simulink model "TosAeb"  (R2021a)
settings: Solver=FixedStepDiscrete  StartTime=0.0  StopTime=inf  FixedStep=0.01  RelTol=1e-3
undefined symbols (not defined in the file or in its folder): ACCModSts, AccSts1, AEB_ObjectMovingOrientation, AEB_ObjectMovingState, CN_BTCActive, CN_CurrentGearR, CN_CurrentGearR_DHT, CN_PTCActive, CN_VDCActive, CN_VSC_EspOff, CN_VSC_FCW_AEB_Error, CN_VSC_FCW_AEB_NoDispay, CN_VSC_FCW_AEB_PerfmDgdtn, CN_VSC_FCW_AEB_TempUnAvlbl, CN_VSC_VehStandstill, CNaccelAvoidMax, CNaccelAvoidMin, CNbrakePedalPress, CNcomfortStrLeftAccelMax, CNcomfortStrLeftAccelMin, CNcomfortStrRightAccelMax, CNcomfortStrRightAccelMin, CNDrvDoorOpen, CNDrvModeEco, CNDrvModeNorm, CNDrvModeOther, CNDrvModeSport, CNDrvSBRreminder, CNNull, CNobjInvalidID, CNPassDoorOpen, CNPassSBRreminder, CNPEBSAccelNegInf, CNPEBSAccelNull, CNPEBSTimeInf, CNPEBSTimeNull, CNSTOPMAX, CNSTOPNULL, CNsubjWidth, CNSwtOFF, CNSwtON, CNTTCMAX, CNTTCNULL, CNVehConfigAEBCycNone, CNVehConfigAEBNone, CNVehConfigAEBPedNone, CNVehConfigFCWNone, CNVehConfigJANone, CollisionSeverity, FALSE, FCWSnvty, JACycObjCalcInfoDefaultParams, JAPedObjCalcInfoDefaultParams, JAVehObjCalcInfoDefaultParams, latCycObjCalcInfoDefaultParams, latPedObjCalcInfoDefaultParams, longObjCalcInfoDefaultParams, ModSts, movingStatus, ObjectType ... (+1112)
libraries: simulink x3031, AEBSFunctionLib x50, Ta_Library x33, AEBSStateMachineLib x23, Mylibrary x12, simulink_extras x7, ObjSelect_lib x3, dspstat3 x2
(part 28 of 102)

== system TosAeb/FcwAebJa_Step_sys/ALG_AEBSystem/ALG_AEBController/AEB_FuncImpManagementApp/FuncImpArbCalc/BrkReadyFuncImpArbCalc1/BrkFuncImpSuppCondCalc ==
  prefillModStsArb : Inport
  ABAModStsArb : Inport  Port=2
  pedPrefillModStsArb : Inport  Port=3
  cycPrefillModStsArb : Inport  Port=4
  JA_vehPrefillModStsArb : Inport  Port=5
  JA_PTW_PrefillModStsArb : Inport  Port=6
  JA_cycPrefillModStsArb : Inport  Port=7
  JA_pedPrefillModStsArb : Inport  Port=8
  brkFuncActiveCond : Inport  Port=9
  Bus Creator : BusCreator  Inputs=9
  Bus Selector : BusSelector  OutputSignals=brkFuncActiveCond
  Bus Selector1 : BusSelector  OutputSignals=AEBModStsArb.latAEBModStsArb.pedABAModStsArb
  Bus Selector10 : BusSelector  OutputSignals=ABAModStsArb,pedABAModStsArb
  Bus Selector2 : BusSelector  OutputSignals=brkFuncActiveCond,AEBModStsArb.vehAEBModStsArb.prefillModStsArb
  Bus Selector3 : BusSelector  OutputSignals=AEBModStsArb.vehAEBModStsArb.prefillModStsArb,AEBModStsArb.latAEBModStsArb.pedPrefillModStsArb,brkFuncActiveCond
  Bus Selector4 : BusSelector  OutputSignals=AEBModStsArb.vehAEBModStsArb.prefillModStsArb,AEBModStsArb.latAEBModStsArb.pedPrefillModStsArb,AEBModStsArb.latAEBModStsArb.cycPrefillModStsArb,brkFuncActiveCond
  Bus Selector5 : BusSelector  OutputSignals=prefillModStsArb,pedPrefillModStsArb,JA_PTW_PrefillModStsArb,JA_cycPrefillModStsArb,JA_pedPrefillModStsArb,brkFuncActiveCond
  Bus Selector6 : BusSelector  OutputSignals=prefillModStsArb,pedPrefillModStsArb,brkFuncActiveCond
  Bus Selector7 : BusSelector  OutputSignals=prefillModStsArb,pedPrefillModStsArb,JA_pedPrefillModStsArb,brkFuncActiveCond
  Bus Selector8 : BusSelector  OutputSignals=prefillModStsArb,pedPrefillModStsArb,JA_cycPrefillModStsArb,JA_pedPrefillModStsArb,brkFuncActiveCond
  Bus Selector9 : BusSelector  OutputSignals=prefillModStsArb,pedPrefillModStsArb,JA_cycPrefillModStsArb,JA_pedPrefillModStsArb,brkFuncActiveCond
  Compare To Constant{,2,4..5,7..8,10..15,17..21,23..24,26..28,30..32,34..35} (27 alike) : simulink/Logic and Bit Operations/Compare To Constant  relop===  const=ModSts.ActiveState  OutDataTypeStr=boolean
  Compare To Constant{1,3,6,9,16,22,25,29,33} (9 alike) : simulink/Logic and Bit Operations/Compare To Constant  relop===  const=TRUE  OutDataTypeStr=boolean
  Constant : Constant  Value=FALSE
  Logical Operator : Logic  Operator=OR  AllPortsSameDT=off  OutDataTypeStr=boolean
  Logical Operator1 : Logic  Operator=OR  Inputs=3  AllPortsSameDT=off  OutDataTypeStr=boolean
  Logical Operator2 : Logic  Operator=OR  Inputs=4  AllPortsSameDT=off  OutDataTypeStr=boolean
  Logical Operator3 : Logic  Operator=OR  Inputs=6  AllPortsSameDT=off  OutDataTypeStr=boolean
  Logical Operator4 : Logic  Operator=OR  Inputs=3  AllPortsSameDT=off  OutDataTypeStr=boolean
  Logical Operator5 : Logic  Operator=OR  Inputs=4  AllPortsSameDT=off  OutDataTypeStr=boolean
  Logical Operator6 : Logic  Operator=OR  Inputs=5  AllPortsSameDT=off  OutDataTypeStr=boolean
  Logical Operator7 : Logic  Operator=OR  Inputs=5  AllPortsSameDT=off  OutDataTypeStr=boolean
  Logical Operator8 : Logic  Operator=OR  AllPortsSameDT=off  OutDataTypeStr=boolean
  JA_PrefillFuncImpSuppCond : Outport
  cycPrefillFuncImpSuppCond : Outport  Port=2
  pedPrefillFuncImpSuppCond : Outport  Port=3
  prefillFuncImpSuppCond : Outport  Port=4
  ABAFuncImpSuppCond : Outport  Port=5
  pedABAFuncImpSuppCond : Outport  Port=6
  JA_vehPrefillFuncImpSuppCond : Outport  Port=7
  JA_pedPrefillFuncImpSuppCond : Outport  Port=8
  JA_cycPrefillFuncImpSuppCond : Outport  Port=9
  JA_PTW_PrefillFuncImpSuppCond : Outport  Port=10
  FCTB_PrefillFuncImpSuppCond : Outport  Port=11
  FCTB_ABAPrefillFuncImpSuppCond : Outport  Port=12
  wires:
  Bus Selector/1 -> Compare To Constant1/1  "<brkFuncActiveCond>"
  Compare To Constant1/1 -> prefillFuncImpSuppCond/1
  Bus Selector1/1 -> Compare To Constant/1  "<pedABAModStsArb>"
  Compare To Constant/1 -> ABAFuncImpSuppCond/1
  Bus Selector2/1 -> Compare To Constant3/1  "<brkFuncActiveCond>"
  Bus Selector2/2 -> Compare To Constant2/1  "<prefillModStsArb>"
  Compare To Constant3/1 -> Logical Operator/1
  Compare To Constant2/1 -> Logical Operator/2
  Logical Operator/1 -> pedPrefillFuncImpSuppCond/1
  Constant/1 -> pedABAFuncImpSuppCond/1
  Bus Selector3/1 -> Compare To Constant4/1  "<prefillModStsArb>"
  Bus Selector3/2 -> Compare To Constant5/1  "<pedPrefillModStsArb>"
  Bus Selector3/3 -> Compare To Constant6/1  "<brkFuncActiveCond>"
  Compare To Constant4/1 -> Logical Operator1/1
  Compare To Constant5/1 -> Logical Operator1/2
  Compare To Constant6/1 -> Logical Operator1/3
  Logical Operator1/1 -> cycPrefillFuncImpSuppCond/1
  Logical Operator2/1 -> JA_PrefillFuncImpSuppCond/1
  Compare To Constant9/1 -> Logical Operator2/4
  Compare To Constant8/1 -> Logical Operator2/2
  Compare To Constant7/1 -> Logical Operator2/1
  Bus Selector4/4 -> Compare To Constant9/1  "<brkFuncActiveCond>"
  Bus Selector4/2 -> Compare To Constant8/1  "<pedPrefillModStsArb>"
  Bus Selector4/1 -> Compare To Constant7/1  "<prefillModStsArb>"
  Bus Selector4/3 -> Compare To Constant10/1  "<cycPrefillModStsArb>"
  Compare To Constant10/1 -> Logical Operator2/3
  JA_pedPrefillModStsArb/1 -> Bus Creator/8  "JA_pedPrefillModStsArb"
  JA_PTW_PrefillModStsArb/1 -> Bus Creator/6  "JA_PTW_PrefillModStsArb"
  JA_cycPrefillModStsArb/1 -> Bus Creator/7  "JA_cycPrefillModStsArb"
  brkFuncActiveCond/1 -> Bus Creator/9  "brkFuncActiveCond"
  prefillModStsArb/1 -> Bus Creator/1  "prefillModStsArb"
  pedPrefillModStsArb/1 -> Bus Creator/3  "pedPrefillModStsArb"
  ABAModStsArb/1 -> Bus Creator/2  "ABAModStsArb"
  JA_vehPrefillModStsArb/1 -> Bus Creator/5  "JA_vehPrefillModStsArb"
  cycPrefillModStsArb/1 -> Bus Creator/4  "cycPrefillModStsArb"
  Compare To Constant31/1 -> Logical Operator7/3
  Compare To Constant30/1 -> Logical Operator7/2
  Bus Selector9/3 -> Compare To Constant31/1  "<JA_cycPrefillModStsArb>"
  Bus Selector9/5 -> Compare To Constant33/1  "<brkFuncActiveCond>"
  Bus Selector9/4 -> Compare To Constant32/1  "<JA_pedPrefillModStsArb>"
  Compare To Constant32/1 -> Logical Operator7/4
  Bus Selector5/1 -> Compare To Constant11/1  "<prefillModStsArb>"
  Compare To Constant12/1 -> Logical Operator3/2
  Bus Selector5/2 -> Compare To Constant12/1  "<pedPrefillModStsArb>"
  Logical Operator6/1 -> JA_PTW_PrefillFuncImpSuppCond/1
  Bus Selector8/5 -> Compare To Constant29/1  "<brkFuncActiveCond>"
  Bus Selector8/2 -> Compare To Constant23/1  "<pedPrefillModStsArb>"
  Bus Selector8/1 -> Compare To Constant21/1  "<prefillModStsArb>"
  Compare To Constant13/1 -> Logical Operator3/3
  Compare To Constant25/1 -> Logical Operator5/4
  Compare To Constant22/1 -> Logical Operator4/3
  Bus Creator/1 -> Bus Selector10/1, Bus Selector9/1, Bus Selector8/1, Bus Selector7/1, Bus Selector6/1, Bus Selector5/1, Bus Selector1/1, Bus Selector/1, Bus Selector2/1, Bus Selector3/1, Bus Selector4/1
  Compare To Constant11/1 -> Logical Operator3/1
  Bus Selector7/2 -> Compare To Constant20/1  "<pedPrefillModStsArb>"
  Bus Selector7/4 -> Compare To Constant25/1  "<brkFuncActiveCond>"
  Compare To Constant33/1 -> Logical Operator7/5
  Compare To Constant28/1 -> Logical Operator6/4
  Bus Selector6/3 -> Compare To Constant22/1  "<brkFuncActiveCond>"
  Compare To Constant20/1 -> Logical Operator5/2
  Compare To Constant14/1 -> Logical Operator3/4
  Logical Operator5/1 -> JA_cycPrefillFuncImpSuppCond/1
  Bus Selector5/5 -> Compare To Constant15/1  "<JA_pedPrefillModStsArb>"
  Bus Selector8/3 -> Compare To Constant27/1  "<JA_cycPrefillModStsArb>"
  Compare To Constant26/1 -> Logical Operator7/1
  Compare To Constant18/1 -> Logical Operator4/2
  Compare To Constant17/1 -> Logical Operator4/1
  Bus Selector5/3 -> Compare To Constant13/1  "<JA_PTW_PrefillModStsArb>"
  Compare To Constant21/1 -> Logical Operator6/1
  Compare To Constant15/1 -> Logical Operator3/5
  Bus Selector5/6 -> Compare To Constant16/1  "<brkFuncActiveCond>"
  Bus Selector7/3 -> Compare To Constant24/1  "<JA_pedPrefillModStsArb>"
  Compare To Constant23/1 -> Logical Operator6/2
  Bus Selector5/4 -> Compare To Constant14/1  "<JA_cycPrefillModStsArb>"
  Compare To Constant16/1 -> Logical Operator3/6
  Bus Selector8/4 -> Compare To Constant28/1  "<JA_pedPrefillModStsArb>"
  Compare To Constant29/1 -> Logical Operator6/5
  Bus Selector9/1 -> Compare To Constant26/1  "<prefillModStsArb>"
  Compare To Constant34/1 -> Logical Operator8/1
  Logical Operator8/1 -> FCTB_ABAPrefillFuncImpSuppCond/1
  Logical Operator7/1 -> FCTB_PrefillFuncImpSuppCond/1
  Compare To Constant35/1 -> Logical Operator8/2
  Logical Operator4/1 -> JA_pedPrefillFuncImpSuppCond/1
  Compare To Constant24/1 -> Logical Operator5/3
  Compare To Constant27/1 -> Logical Operator6/3
  Bus Selector10/2 -> Compare To Constant35/1  "<pedABAModStsArb>"
  Logical Operator3/1 -> JA_vehPrefillFuncImpSuppCond/1
  Bus Selector9/2 -> Compare To Constant30/1  "<pedPrefillModStsArb>"
  Bus Selector6/1 -> Compare To Constant17/1  "<prefillModStsArb>"
  Bus Selector7/1 -> Compare To Constant19/1  "<prefillModStsArb>"
  Bus Selector10/1 -> Compare To Constant34/1  "<ABAModStsArb>"
  Bus Selector6/2 -> Compare To Constant18/1  "<pedPrefillModStsArb>"
  Compare To Constant19/1 -> Logical Operator5/1

== system TosAeb/FcwAebJa_Step_sys/ALG_AEBSystem/ALG_AEBController/AEB_FuncImpManagementApp/FuncImpArbCalc/BrkReadyFuncImpArbCalc1/BrkReadyFuncActvCondCalc ==
  PrefillFuncImpArb : Inport
  ABAFuncImpArb : Inport  Port=2
  pedPrefillFuncImpArb : Inport  Port=3
  pedABAFuncImpArb : Inport  Port=4
  cycPrefillFuncImpArb : Inport  Port=5
  JA_vehPrefillFuncImpArb : Inport  Port=6
  Compare To Constant{,1..5} (6 alike) : simulink/Logic and Bit Operations/Compare To Constant  relop===  const=ModSts.ActiveState  OutDataTypeStr=boolean
  Logical Operator : Logic  Operator=OR  Inputs=6  AllPortsSameDT=off  OutDataTypeStr=boolean
  brkReadyActvCond : Outport
  wires:
  PrefillFuncImpArb/1 -> Compare To Constant/1
  ABAFuncImpArb/1 -> Compare To Constant1/1
  pedPrefillFuncImpArb/1 -> Compare To Constant2/1
  pedABAFuncImpArb/1 -> Compare To Constant3/1
  cycPrefillFuncImpArb/1 -> Compare To Constant4/1
  JA_vehPrefillFuncImpArb/1 -> Compare To Constant5/1
  Compare To Constant4/1 -> Logical Operator/5
  Compare To Constant5/1 -> Logical Operator/6
  Compare To Constant3/1 -> Logical Operator/4
  Compare To Constant2/1 -> Logical Operator/3
  Compare To Constant1/1 -> Logical Operator/2
  Compare To Constant/1 -> Logical Operator/1
  Logical Operator/1 -> brkReadyActvCond/1

== system TosAeb/FcwAebJa_Step_sys/ALG_AEBSystem/ALG_AEBController/AEB_FuncImpManagementApp/FuncImpArbCalc/BrkReadyFuncImpArbCalc1/BrkReadyFuncImpAborCondCalc ==
  prefillModStsArb : Inport
  ABAModStsArb : Inport  Port=2
  pedPrefillModStsArb : Inport  Port=3
  pedABAModStsArb : Inport  Port=4
  cycPrefillModStsArb : Inport  Port=5
  JA_vehPrefillModStsArb : Inport  Port=6
  JA_PTW_PrefillModStsArb : Inport  Port=7
  JA_cycPrefillModStsArb : Inport  Port=8
  JA_pedPrefillModStsArb : Inport  Port=9
  brkFuncActiveCond : Inport  Port=10
  Bus Creator : BusCreator  Inputs=10
  Bus Selector : BusSelector  OutputSignals=brkFuncActiveCond
  Bus Selector1 : BusSelector  OutputSignals=prefillModStsArb,brkFuncActiveCond
  Bus Selector10 : BusSelector  OutputSignals=ABAModStsArb,pedABAModStsArb
  Bus Selector2 : BusSelector  OutputSignals=prefillModStsArb,pedPrefillModStsArb,brkFuncActiveCond
  Bus Selector3 : BusSelector  OutputSignals=prefillModStsArb,pedPrefillModStsArb,cycPrefillModStsArb,brkFuncActiveCond
  Bus Selector4 : BusSelector  OutputSignals=pedPrefillModStsArb
  Bus Selector5 : BusSelector  OutputSignals=prefillModStsArb,pedPrefillModStsArb,JA_PTW_PrefillModStsArb,JA_cycPrefillModStsArb,JA_pedPrefillModStsArb,brkFuncActiveCond
  Bus Selector6 : BusSelector  OutputSignals=prefillModStsArb,pedPrefillModStsArb,brkFuncActiveCond
  Bus Selector7 : BusSelector  OutputSignals=prefillModStsArb,pedPrefillModStsArb,JA_pedPrefillModStsArb,brkFuncActiveCond
  Bus Selector8 : BusSelector  OutputSignals=prefillModStsArb,pedPrefillModStsArb,JA_cycPrefillModStsArb,JA_pedPrefillModStsArb,brkFuncActiveCond
  Bus Selector9 : BusSelector  OutputSignals=prefillModStsArb,pedPrefillModStsArb,JA_cycPrefillModStsArb,JA_pedPrefillModStsArb,brkFuncActiveCond
  Compare To Constant{,3,6,9,16,22,25,29,33} (9 alike) : simulink/Logic and Bit Operations/Compare To Constant  relop===  const=TRUE  OutDataTypeStr=boolean
  Compare To Constant{1..2,4..5,7..8,10..15,17..21,23..24,26..28,30..32,34..35} (27 alike) : simulink/Logic and Bit Operations/Compare To Constant  relop===  const=ModSts.ActiveState  OutDataTypeStr=boolean
  Constant : Constant  Value=FALSE
  Logical Operator : Logic  Operator=OR  AllPortsSameDT=off  OutDataTypeStr=boolean
  Logical Operator1 : Logic  Operator=OR  Inputs=3  AllPortsSameDT=off  OutDataTypeStr=boolean
  Logical Operator2 : Logic  Operator=OR  Inputs=4  AllPortsSameDT=off  OutDataTypeStr=boolean
  Logical Operator3 : Logic  Operator=OR  Inputs=6  AllPortsSameDT=off  OutDataTypeStr=boolean
  Logical Operator4 : Logic  Operator=OR  Inputs=3  AllPortsSameDT=off  OutDataTypeStr=boolean
  Logical Operator5 : Logic  Operator=OR  Inputs=4  AllPortsSameDT=off  OutDataTypeStr=boolean
  Logical Operator6 : Logic  Operator=OR  Inputs=5  AllPortsSameDT=off  OutDataTypeStr=boolean
  Logical Operator7 : Logic  Operator=OR  Inputs=5  AllPortsSameDT=off  OutDataTypeStr=boolean
  Logical Operator8 : Logic  Operator=OR  AllPortsSameDT=off  OutDataTypeStr=boolean
  JA_vehPrefillFuncImpAborCond : Outport
  cycPrefillFuncImpAborCond : Outport  Port=2
  pedPrefillFuncImpAborCond : Outport  Port=3
  prefillFuncImpAborCond : Outport  Port=4
  ABAFuncImpAborCond : Outport  Port=5
  pedABAFuncImpAborCond : Outport  Port=6
  JA_vehPrefillFuncImpAborArb : Outport  Port=7
  JA_pedPrefillFuncImpAborArb : Outport  Port=8
  JA_cycPrefillFuncImpAborArb : Outport  Port=9
  JA_PTW_PrefillFuncImpAborArb : Outport  Port=10
  FCTB_PrefillFuncImpAborArb : Outport  Port=11
  FCTB_ABAPrefillFuncImpAborArb : Outport  Port=12
  wires:
  Compare To Constant/1 -> prefillFuncImpAborCond/1
  Compare To Constant2/1 -> Logical Operator/1
  Compare To Constant3/1 -> Logical Operator/2
  Logical Operator/1 -> pedPrefillFuncImpAborCond/1
  Constant/1 -> pedABAFuncImpAborCond/1
  Compare To Constant4/1 -> Logical Operator1/1
  Compare To Constant6/1 -> Logical Operator1/3
  Compare To Constant5/1 -> Logical Operator1/2
  Logical Operator1/1 -> cycPrefillFuncImpAborCond/1
  Logical Operator2/1 -> JA_vehPrefillFuncImpAborCond/1
  Compare To Constant8/1 -> Logical Operator2/2
  Compare To Constant9/1 -> Logical Operator2/4
  Compare To Constant7/1 -> Logical Operator2/1
  Compare To Constant10/1 -> Logical Operator2/3
  ABAModStsArb/1 -> Bus Creator/2  "ABAModStsArb"
  prefillModStsArb/1 -> Bus Creator/1  "prefillModStsArb"
  pedPrefillModStsArb/1 -> Bus Creator/3  "pedPrefillModStsArb"
  cycPrefillModStsArb/1 -> Bus Creator/5  "cycPrefillModStsArb"
  JA_vehPrefillModStsArb/1 -> Bus Creator/6  "JA_vehPrefillModStsArb"
  brkFuncActiveCond/1 -> Bus Creator/10  "brkFuncActiveCond"
  Bus Selector/1 -> Compare To Constant/1  "<brkFuncActiveCond>"
  Bus Selector1/1 -> Compare To Constant2/1  "<prefillModStsArb>"
  Bus Selector1/2 -> Compare To Constant3/1  "<brkFuncActiveCond>"
  Bus Selector2/3 -> Compare To Constant6/1  "<brkFuncActiveCond>"
  Bus Selector2/2 -> Compare To Constant5/1  "<pedPrefillModStsArb>"
  Bus Selector2/1 -> Compare To Constant4/1  "<prefillModStsArb>"
  Bus Selector3/2 -> Compare To Constant8/1  "<pedPrefillModStsArb>"
  Bus Selector3/1 -> Compare To Constant7/1  "<prefillModStsArb>"
  Bus Selector3/3 -> Compare To Constant10/1  "<cycPrefillModStsArb>"
  Bus Selector3/4 -> Compare To Constant9/1  "<brkFuncActiveCond>"
  Bus Selector4/1 -> Compare To Constant11/1  "<pedPrefillModStsArb>"
  Compare To Constant11/1 -> ABAFuncImpAborCond/1
  JA_PTW_PrefillModStsArb/1 -> Bus Creator/7  "JA_PTW_PrefillModStsArb"
  JA_cycPrefillModStsArb/1 -> Bus Creator/8  "JA_cycPrefillModStsArb"
  JA_pedPrefillModStsArb/1 -> Bus Creator/9  "JA_pedPrefillModStsArb"
  pedABAModStsArb/1 -> Bus Creator/4  "pedABAModStsArb"
  Bus Selector5/1 -> Compare To Constant1/1  "<prefillModStsArb>"
  Bus Selector5/2 -> Compare To Constant12/1  "<pedPrefillModStsArb>"
  Bus Selector5/3 -> Compare To Constant13/1  "<JA_PTW_PrefillModStsArb>"
  Bus Selector5/4 -> Compare To Constant14/1  "<JA_cycPrefillModStsArb>"
  Bus Selector5/5 -> Compare To Constant15/1  "<JA_pedPrefillModStsArb>"
  Bus Selector5/6 -> Compare To Constant16/1  "<brkFuncActiveCond>"
  Compare To Constant16/1 -> Logical Operator3/6
  Compare To Constant15/1 -> Logical Operator3/5
  Compare To Constant14/1 -> Logical Operator3/4
  Compare To Constant13/1 -> Logical Operator3/3
  Compare To Constant12/1 -> Logical Operator3/2
  Compare To Constant1/1 -> Logical Operator3/1
  Logical Operator3/1 -> JA_vehPrefillFuncImpAborArb/1
  Logical Operator4/1 -> JA_pedPrefillFuncImpAborArb/1
  Compare To Constant17/1 -> Logical Operator4/1
  Compare To Constant18/1 -> Logical Operator4/2
  Compare To Constant22/1 -> Logical Operator4/3
  Bus Selector6/3 -> Compare To Constant22/1  "<brkFuncActiveCond>"
  Bus Selector6/2 -> Compare To Constant18/1  "<pedPrefillModStsArb>"
  Bus Selector6/1 -> Compare To Constant17/1  "<prefillModStsArb>"
  Logical Operator5/1 -> JA_cycPrefillFuncImpAborArb/1
  Compare To Constant19/1 -> Logical Operator5/1
  Compare To Constant20/1 -> Logical Operator5/2
  Compare To Constant24/1 -> Logical Operator5/3
  Compare To Constant25/1 -> Logical Operator5/4
  Bus Selector7/4 -> Compare To Constant25/1  "<brkFuncActiveCond>"
  Bus Selector7/3 -> Compare To Constant24/1  "<JA_pedPrefillModStsArb>"
  Bus Selector7/2 -> Compare To Constant20/1  "<pedPrefillModStsArb>"
  Bus Selector7/1 -> Compare To Constant19/1  "<prefillModStsArb>"
  Compare To Constant27/1 -> Logical Operator6/3
  Bus Selector8/1 -> Compare To Constant21/1  "<prefillModStsArb>"
  Compare To Constant23/1 -> Logical Operator6/2
  Bus Selector8/2 -> Compare To Constant23/1  "<pedPrefillModStsArb>"
  Bus Selector8/3 -> Compare To Constant27/1  "<JA_cycPrefillModStsArb>"
  Compare To Constant21/1 -> Logical Operator6/1
  Compare To Constant29/1 -> Logical Operator6/5
  Bus Selector8/4 -> Compare To Constant28/1  "<JA_pedPrefillModStsArb>"
  Bus Selector8/5 -> Compare To Constant29/1  "<brkFuncActiveCond>"
  Compare To Constant28/1 -> Logical Operator6/4
  Logical Operator6/1 -> JA_PTW_PrefillFuncImpAborArb/1
  Logical Operator7/1 -> FCTB_PrefillFuncImpAborArb/1
  Compare To Constant32/1 -> Logical Operator7/4
  Bus Selector9/5 -> Compare To Constant33/1  "<brkFuncActiveCond>"
  Bus Selector9/4 -> Compare To Constant32/1  "<JA_pedPrefillModStsArb>"
  Compare To Constant33/1 -> Logical Operator7/5
  Compare To Constant26/1 -> Logical Operator7/1
  Bus Selector9/3 -> Compare To Constant31/1  "<JA_cycPrefillModStsArb>"
  Bus Selector9/2 -> Compare To Constant30/1  "<pedPrefillModStsArb>"
  Compare To Constant30/1 -> Logical Operator7/2
  Bus Selector9/1 -> Compare To Constant26/1  "<prefillModStsArb>"
  Compare To Constant31/1 -> Logical Operator7/3
  Bus Creator/1 -> Bus Selector10/1, Bus Selector9/1, Bus Selector8/1, Bus Selector7/1, Bus Selector6/1, Bus Selector5/1, Bus Selector4/1, Bus Selector/1, Bus Selector1/1, Bus Selector2/1, Bus Selector3/1
  Bus Selector10/1 -> Compare To Constant34/1  "<ABAModStsArb>"
  Bus Selector10/2 -> Compare To Constant35/1  "<pedABAModStsArb>"
  Compare To Constant35/1 -> Logical Operator8/2
  Compare To Constant34/1 -> Logical Operator8/1
  Logical Operator8/1 -> FCTB_ABAPrefillFuncImpAborArb/1

== system TosAeb/FcwAebJa_Step_sys/ALG_AEBSystem/ALG_AEBController/AEB_FuncImpManagementApp/FuncImpArbCalc/BrkReadyFuncImpArbCalc1/BrkReadyFuncImpArbCalc ==
  prefillModStsArb : Inport
  ABAModStsArb : Inport  Port=2
  pedPrefillModStsArb : Inport  Port=3
  cycPrefillModStsArb : Inport  Port=4
  JA_vehPrefillModStsArb : Inport  Port=5
  JA_PTW_PrefillModStsArb : Inport  Port=6
  JA_cycPrefillModStsArb : Inport  Port=7
  JA_pedPrefillModStsArb : Inport  Port=8
  JA_vehPrefillFuncImpAborCond : Inport  Port=9
  cycPrefillFuncImpAborCond : Inport  Port=10
  pedPrefillFuncImpAborCond : Inport  Port=11
  vehPrefillFuncImpAborCond : Inport  Port=12
  vehABAFuncImpAborCond : Inport  Port=13
  pedABAFuncImpAborCond : Inport  Port=14
  JA_vehPrefillFuncImpAborArb : Inport  Port=15
  JA_pedPrefillFuncImpAborArb : Inport  Port=16
  JA_cycPrefillFuncImpAborArb : Inport  Port=17
  JA_PTW_PrefillFuncImpAborArb : Inport  Port=18
  FCTB_PrefillFuncImpAborArb : Inport  Port=19
  FCTB_ABAPrefillFuncImpAborArb : Inport  Port=20
  ABAFuncImpArbCalc : Subsystem
  Bus Creator : BusCreator  Inputs=2
  Bus Creator4 : BusCreator  Inputs=8
  Bus Selector : BusSelector  OutputSignals=AEBModStsArb.vehAEBModStsArb.prefillModStsArb,brkReadyFuncImpAborCond.vehPrefillFuncImpAborCond
  Bus Selector1 : BusSelector  OutputSignals=AEBModStsArb.vehAEBModStsArb.ABAModStsArb,brkReadyFuncImpAborCond.vehABAFuncImpAborCond
  Bus Selector2 : BusSelector  OutputSignals=AEBModStsArb.latAEBModStsArb.pedPrefillModStsArb,brkReadyFuncImpAborCond.pedPrefillFuncImpAborCond
  Bus Selector3 : BusSelector  OutputSignals=AEBModStsArb.latAEBModStsArb.pedABAModStsArb,brkReadyFuncImpAborCond.pedABAFuncImpAborCond
  Bus Selector4 : BusSelector  OutputSignals=AEBModStsArb.latAEBModStsArb.cycPrefillModStsArb,brkReadyFuncImpAborCond.cycPrefillFuncImpAborCond
  Bus Selector5 : BusSelector  OutputSignals=AEBModStsArb.JA_vehAEBModStsArb.JA_vehPrefillModStsArb,brkReadyFuncImpAborCond.JA_vehPrefillFuncImpAborCond
  JA_vehPrefillFuncImpArbCalc : Subsystem
  PrefillFuncImpArbCalc : Subsystem
  cycPrefillFuncImpArbCalc : Subsystem
  pedABAFuncImpArbCalc : Subsystem
  pedPrefillFuncImpArbCalc : Subsystem
  prefillFuncImpArb : Outport
  ABAFuncImpArb : Outport  Port=2
  pedPrefillFuncImpArb : Outport  Port=3
  pedABAFuncImpArb : Outport  Port=4
  cycPrefillFuncImpArb : Outport  Port=5
  JA_vehPrefillFuncImpArb : Outport  Port=6
  wires:
  Bus Creator4/1 -> Bus Creator/1  "AEBModStsArb"
  Bus Creator/1 -> Bus Selector5/1, Bus Selector4/1, Bus Selector3/1, Bus Selector2/1, Bus Selector1/1, Bus Selector/1
  Bus Selector/1 -> PrefillFuncImpArbCalc/1(prefillModStsArb)  "<prefillModStsArb>"
  Bus Selector/2 -> PrefillFuncImpArbCalc/2(prefillFuncImpAborCon d)  "<vehPrefillFuncImpAborCond>"
  Bus Selector1/1 -> ABAFuncImpArbCalc/1(ABAModStsArb)  "<ABAModStsArb>"
  Bus Selector1/2 -> ABAFuncImpArbCalc/2(ABAFuncImpAborCond)  "<vehABAFuncImpAborCond>"
  Bus Selector2/1 -> pedPrefillFuncImpArbCalc/1(pedPrefillModStsArb)  "<pedPrefillModStsArb>"
  Bus Selector2/2 -> pedPrefillFuncImpArbCalc/2(pedPrefillFuncImpAborCond)  "<pedPrefillFuncImpAborCond>"
  Bus Selector3/1 -> pedABAFuncImpArbCalc/1(pedABAModStsArb)  "<pedABAModStsArb>"
  Bus Selector3/2 -> pedABAFuncImpArbCalc/2(pedABAFuncImpAborCond)  "<pedABAFuncImpAborCond>"
  Bus Selector4/1 -> cycPrefillFuncImpArbCalc/1(cycPrefillModStsArb)  "<cycPrefillModStsArb>"
  Bus Selector4/2 -> cycPrefillFuncImpArbCalc/2(cycPrefillFuncImpAborCond)  "<cycPrefillFuncImpAborCond>"
  Bus Selector5/1 -> JA_vehPrefillFuncImpArbCalc/1(JA_vehPrefillModStsArb)  "<JA_vehPrefillModStsArb>"
  Bus Selector5/2 -> JA_vehPrefillFuncImpArbCalc/2(JA_vehPrefillFuncImpAborCond)  "<JA_vehPrefillFuncImpAborCond>"
  PrefillFuncImpArbCalc/1(prefillFuncImpArb) -> prefillFuncImpArb/1  "prefillFuncImpArb"
  ABAFuncImpArbCalc/1(ABAFuncImpArb) -> ABAFuncImpArb/1  "ABAFuncImpArb"
  JA_vehPrefillFuncImpArbCalc/1(JA_vehPrefillFuncImpArb) -> JA_vehPrefillFuncImpArb/1  "JA_vehPrefillFuncImpArb"
  cycPrefillFuncImpArbCalc/1(cycPrefillFuncImpArb) -> cycPrefillFuncImpArb/1  "cycPrefillFuncImpArb"
  pedABAFuncImpArbCalc/1(pedABAFuncImpArb) -> pedABAFuncImpArb/1  "pedABAFuncImpArb"
  pedPrefillFuncImpArbCalc/1(pedPrefillFuncImpArb) -> pedPrefillFuncImpArb/1  "pedPrefillFuncImpArb"
  JA_cycPrefillModStsArb/1 -> Bus Creator4/7  "JA_cycPrefillModStsArb"
  prefillModStsArb/1 -> Bus Creator4/1  "prefillModStsArb"
  JA_vehPrefillModStsArb/1 -> Bus Creator4/5  "JA_vehPrefillModStsArb"
  pedPrefillModStsArb/1 -> Bus Creator4/3  "pedPrefillModStsArb"
  ABAModStsArb/1 -> Bus Creator4/2  "ABAModStsArb"
  JA_PTW_PrefillModStsArb/1 -> Bus Creator4/6  "JA_PTW_PrefillModStsArb"
  cycPrefillModStsArb/1 -> Bus Creator4/4  "cycPrefillModStsArb"
  JA_pedPrefillModStsArb/1 -> Bus Creator4/8  "JA_pedPrefillModStsArb"

== system TosAeb/FcwAebJa_Step_sys/ALG_AEBSystem/ALG_AEBController/AEB_FuncImpManagementApp/FuncImpArbCalc/WarningFuncImpArbCalc/WarningFuncImpAborCond ==
  AEBModStsArb : Inport
  brkReadyFuncActvCond : Inport  Port=2
  brkFuncActvCond : Inport  Port=3
  Bus Creator : BusCreator  Inputs=3
  Bus Selector : BusSelector  OutputSignals=brkReadyFuncActvCond,brkFuncActvCond,AEBModStsArb.vehAEBModStsArb.preWarningModStsArb,AEBModStsArb.vehAEBModStsArb.acuteWarningModStsArb,AEBModStsArb.latAEBModStsArb.pedPreWarningModStsArb
  Bus Selector1 : BusSelector  OutputSignals=AEBModStsArb.latAEBModStsArb.pedAEBfullModStsArb,AEBModStsArb.latAEBModStsArb.cycAEBfullModStsArb,AEBModStsArb.vehAEBModStsArb.EBAModStsArb
  Bus Selector2 : BusSelector  OutputSignals=brkFuncActvCond
  Bus Selector3 : BusSelector  OutputSignals=AEBModStsArb.vehAEBModStsArb.preWarningModStsArb,AEBModStsArb.latAEBModStsArb.cycAEBfullModStsArb,AEBModStsArb.vehAEBModStsArb.EBAModStsArb,AEBModStsArb.vehAEBModStsArb.AEBfullModStsArb
  Compare To Constant1 : simulink/Logic and Bit Operations/Compare To Constant  relop===  const=TRUE  OutDataTypeStr=boolean
  Compare To Constant{2,5..11,13..14} (10 alike) : simulink/Logic and Bit Operations/Compare To Constant  relop===  const=ModSts.ActiveState  OutDataTypeStr=boolean
  Compare To Constant3 : simulink/Logic and Bit Operations/Compare To Constant  relop===  const=TRUE  OutDataTypeStr=boolean
  Compare To Constant4 : simulink/Logic and Bit Operations/Compare To Constant  relop===  const=TRUE  OutDataTypeStr=boolean
  Logical Operator : Logic  Operator=OR  Inputs=5  AllPortsSameDT=off  OutDataTypeStr=boolean
  Logical Operator1 : Logic  Operator=OR  Inputs=3  AllPortsSameDT=off  OutDataTypeStr=boolean
  Logical Operator2 : Logic  Operator=OR  Inputs=4  AllPortsSameDT=off  OutDataTypeStr=boolean
  latentWarningFuncImpAborCond : Outport
  pedWarningFuncImpAborCond : Outport  Port=2
  preWarningFuncImpAborCond : Outport  Port=3
  acuteWarningFuncImpAborCond : Outport  Port=4
  wires:
  Compare To Constant4/1 -> acuteWarningFuncImpAborCond/1
  Bus Selector2/1 -> Compare To Constant4/1  "<brkFuncActvCond>"
  Logical Operator2/1 -> pedWarningFuncImpAborCond/1
  Compare To Constant3/1 -> Logical Operator/1
  Bus Selector/3 -> Compare To Constant2/1  "<preWarningModStsArb>"
  Bus Selector/4 -> Compare To Constant5/1  "<acuteWarningModStsArb>"
  Bus Selector/1 -> Compare To Constant3/1  "<brkReadyFuncActvCond>"
  Logical Operator/1 -> latentWarningFuncImpAborCond/1
  Compare To Constant2/1 -> Logical Operator/3
  Compare To Constant5/1 -> Logical Operator/4
  Compare To Constant6/1 -> Logical Operator/5
  Bus Selector/5 -> Compare To Constant6/1  "<pedPreWarningModStsArb>"
  Compare To Constant8/1 -> Logical Operator1/3
  Compare To Constant9/1 -> Logical Operator1/1
  Bus Selector1/2 -> Compare To Constant7/1  "<cycAEBfullModStsArb>"
  Bus Selector1/3 -> Compare To Constant8/1  "<EBAModStsArb>"
  Compare To Constant7/1 -> Logical Operator1/2
  Bus Selector1/1 -> Compare To Constant9/1  "<pedAEBfullModStsArb>"
  Bus Selector/2 -> Compare To Constant1/1  "<brkFuncActvCond>"
  Bus Selector3/3 -> Compare To Constant14/1  "<EBAModStsArb>"
  Bus Selector3/2 -> Compare To Constant13/1  "<cycAEBfullModStsArb>"
  Bus Selector3/4 -> Compare To Constant10/1  "<AEBfullModStsArb>"
  Compare To Constant11/1 -> Logical Operator2/1
  Compare To Constant13/1 -> Logical Operator2/2
  Compare To Constant14/1 -> Logical Operator2/3
  Bus Selector3/1 -> Compare To Constant11/1  "<preWarningModStsArb>"
  Compare To Constant10/1 -> Logical Operator2/4
  AEBModStsArb/1 -> Bus Creator/1  "AEBModStsArb"
  Compare To Constant1/1 -> Logical Operator/2
  brkReadyFuncActvCond/1 -> Bus Creator/2  "brkReadyFuncActvCond"
  brkFuncActvCond/1 -> Bus Creator/3  "brkFuncActvCond"
  Logical Operator1/1 -> preWarningFuncImpAborCond/1
  Bus Creator/1 -> Bus Selector/1, Bus Selector3/1, Bus Selector1/1, Bus Selector2/1

== system TosAeb/FcwAebJa_Step_sys/ALG_AEBSystem/ALG_AEBController/AEB_FuncImpManagementApp/FuncImpArbCalc/WarningFuncImpArbCalc/WarningFuncImpArbCalc ==
  AEBModStsArb : Inport
  warningFuncImpAborCondCond : Inport  Port=2
  AcuteFuncImpArbCalc : Subsystem
  Bus Creator : BusCreator  Inputs=2
  Bus Selector : BusSelector  OutputSignals=AEBModStsArb.vehAEBModStsArb.latentWarningModStsArb,warningFuncImpAborCond.vehLatentWarningFuncImpAborCond
  Bus Selector1 : BusSelector  OutputSignals=AEBModStsArb.vehAEBModStsArb.preWarningModStsArb,warningFuncImpAborCond.vehPreWarningFuncImpAborCond
  Bus Selector2 : BusSelector  OutputSignals=AEBModStsArb.vehAEBModStsArb.acuteWarningModStsArb,warningFuncImpAborCond.vehAcuteWarningFuncimpAborCond
  Bus Selector3 : BusSelector  OutputSignals=AEBModStsArb.latAEBModStsArb.pedPreWarningModStsArb,warningFuncImpAborCond.pedPreWarningFuncImpAborCond
  LatentWarningFuncImpArbCalc : Subsystem
  PreWarningFuncImpArbCalc : Subsystem
  pedPreWarningFuncImpArbCalc : Subsystem
  latentWarningFuncImpArb : Outport
  preWarningFuncImpArb : Outport  Port=2
  acuteWarningFuncImpArb : Outport  Port=3
  pedPreWarningFuncImpArb : Outport  Port=4
  wires:
  pedPreWarningFuncImpArbCalc/1(pedPreWarningFuncImpArb) -> pedPreWarningFuncImpArb/1  "pedPreWarningFuncImpArb"
  AcuteFuncImpArbCalc/1(acuteWarningFuncImpArb) -> acuteWarningFuncImpArb/1  "acuteWarningFuncImpArb"
  PreWarningFuncImpArbCalc/1(preWarningFuncImpArb) -> preWarningFuncImpArb/1  "preWarningFuncImpArb"
  LatentWarningFuncImpArbCalc/1(latentWarningFuncImpArb) -> latentWarningFuncImpArb/1  "latentWarningFuncImpArb"
  Bus Selector3/1 -> pedPreWarningFuncImpArbCalc/1(pedPreWarningModStsArb)  "<pedPreWarningModStsArb>"
  Bus Selector3/2 -> pedPreWarningFuncImpArbCalc/2(pedPreWarningFuncImpAborCond)  "<pedPreWarningFuncImpAborCond>"
  Bus Selector2/2 -> AcuteFuncImpArbCalc/2(acuteWarningFuncImpAborCond)  "<vehAcuteWarningFuncimpAborCond>"
  Bus Selector2/1 -> AcuteFuncImpArbCalc/1(acuteWarningModStsArb)  "<acuteWarningModStsArb>"
  Bus Selector1/1 -> PreWarningFuncImpArbCalc/1(preWarningModStsArb)  "<preWarningModStsArb>"
  Bus Selector1/2 -> PreWarningFuncImpArbCalc/2(preWarningFuncImpAborCond)  "<vehPreWarningFuncImpAborCond>"
  Bus Selector/2 -> LatentWarningFuncImpArbCalc/2(latentWarningFuncImpAborCond)  "<vehLatentWarningFuncImpAborCond>"
  Bus Selector/1 -> LatentWarningFuncImpArbCalc/1(latentWarningModStsArb)  "<latentWarningModStsArb>"
  Bus Creator/1 -> Bus Selector/1, Bus Selector1/1, Bus Selector2/1, Bus Selector3/1
  warningFuncImpAborCondCond/1 -> Bus Creator/2  "warningFuncImpAborCond"
  AEBModStsArb/1 -> Bus Creator/1  "AEBModStsArb"

== system TosAeb/FcwAebJa_Step_sys/ALG_AEBSystem/ALG_AEBController/AEB_FuncImpManagementApp/FuncImpArbCalc/WarningFuncImpArbCalc/WarningFuncImpSuppCond ==
  brkFuncActvCond : Inport
  brkReadyFuncActvCond : Inport  Port=2
  AEBModStsArb : Inport  Port=3
  Bus Creator : BusCreator  Inputs=3
  Bus Selector : BusSelector  OutputSignals=brkReadyFuncActvCond,brkFuncActvCond,AEBModStsArb.vehAEBModStsArb.preWarningModStsArb,AEBModStsArb.vehAEBModStsArb.acuteWarningModStsArb,AEBModStsArb.latAEBModStsArb.pedPreWarningModStsArb
  Bus Selector2 : BusSelector  OutputSignals=brkFuncActvCond
  Bus Selector3 : BusSelector  OutputSignals=brkFuncActvCond,AEBModStsArb.vehAEBModStsArb.preWarningModStsArb,AEBModStsArb.vehAEBModStsArb.acuteWarningModStsArb
  Compare To Constant{1,3..4,11} (4 alike) : simulink/Logic and Bit Operations/Compare To Constant  relop===  const=TRUE  OutDataTypeStr=boolean
  Compare To Constant{2,5..6,13..14} (5 alike) : simulink/Logic and Bit Operations/Compare To Constant  relop===  const=ModSts.ActiveState  OutDataTypeStr=boolean
  Logical Operator : Logic  Operator=OR  Inputs=5  AllPortsSameDT=off  OutDataTypeStr=boolean
  Logical Operator2 : Logic  Operator=OR  Inputs=3  AllPortsSameDT=off  OutDataTypeStr=boolean
  latentWarningFuncImpSuppCond : Outport
  pedWarningFuncImpSuppCond : Outport  Port=2
  preWarningFuncImpSuppCond : Outport  Port=3
  acuteWarningFuncImpSuppCond : Outport  Port=4
  wires:
  Bus Selector3/1 -> Compare To Constant11/1  "<brkFuncActvCond>"
  Bus Selector3/2 -> Compare To Constant13/1  "<preWarningModStsArb>"
  Bus Selector3/3 -> Compare To Constant14/1  "<acuteWarningModStsArb>"
  AEBModStsArb/1 -> Bus Creator/3  "AEBModStsArb"
  brkReadyFuncActvCond/1 -> Bus Creator/2  "brkReadyFuncActvCond"
  brkFuncActvCond/1 -> Bus Creator/1  "brkFuncActvCond"
  Bus Creator/1 -> Bus Selector3/1, Bus Selector2/1, Bus Selector/1
  Compare To Constant1/1 -> Logical Operator/2
  Compare To Constant14/1 -> Logical Operator2/3
  Compare To Constant13/1 -> Logical Operator2/2
  Compare To Constant11/1 -> Logical Operator2/1
  Bus Selector/2 -> Compare To Constant1/1  "<brkFuncActvCond>"
  Bus Selector/5 -> Compare To Constant6/1  "<pedPreWarningModStsArb>"
  Compare To Constant6/1 -> Logical Operator/5
  Compare To Constant5/1 -> Logical Operator/4
  Compare To Constant2/1 -> Logical Operator/3
  Logical Operator/1 -> latentWarningFuncImpSuppCond/1
  Bus Selector/1 -> Compare To Constant3/1  "<brkReadyFuncActvCond>"
  Bus Selector/4 -> Compare To Constant5/1  "<acuteWarningModStsArb>"
  Bus Selector/3 -> Compare To Constant2/1  "<preWarningModStsArb>"
  Compare To Constant3/1 -> Logical Operator/1
  Logical Operator2/1 -> pedWarningFuncImpSuppCond/1
  Bus Selector2/1 -> Compare To Constant4/1  "<brkFuncActvCond>"
  Compare To Constant4/1 -> preWarningFuncImpSuppCond/1, acuteWarningFuncImpSuppCond/1

== system TosAeb/FcwAebJa_Step_sys/ALG_AEBSystem/ALG_AEBController/AEB_FuncImpManagementApp/FuncImpArbCalc/WarningFuncImpArbCalc/warningFuncActvCondCalc ==
  latentWarningFuncImpArb : Inport
  preWarningFuncImpArb : Inport  Port=2
  acuteWarningFuncImpArb : Inport  Port=3
  pedPreWarningFuncImpArb : Inport  Port=4
  Compare To Constant{,1..3} (4 alike) : simulink/Logic and Bit Operations/Compare To Constant  relop===  const=ModSts.ActiveState  OutDataTypeStr=boolean
  Logical Operator : Logic  Operator=OR  Inputs=4  AllPortsSameDT=off  OutDataTypeStr=boolean
  warningFuncActvCond : Outport
  wires:
  Logical Operator/1 -> warningFuncActvCond/1
  Compare To Constant3/1 -> Logical Operator/4
  Compare To Constant2/1 -> Logical Operator/3
  Compare To Constant1/1 -> Logical Operator/2
  Compare To Constant/1 -> Logical Operator/1
  pedPreWarningFuncImpArb/1 -> Compare To Constant3/1
  acuteWarningFuncImpArb/1 -> Compare To Constant2/1
  preWarningFuncImpArb/1 -> Compare To Constant1/1
  latentWarningFuncImpArb/1 -> Compare To Constant/1

== system TosAeb/FcwAebJa_Step_sys/ALG_AEBSystem/ALG_AEBController/AEB_FuncImpManagementApp/FuncManeuArbCalc/BrkReadyManeuArbCalc/BrkReadyManeuAbor ==
  ABPTrigger : Inport
  ABATrigger : Inport  Port=2
  ABPActive : Inport  Port=3
  ABAActive : Inport  Port=4
  Compare To Constant : simulink/Logic and Bit Operations/Compare To Constant  relop===  const=TRUE  OutDataTypeStr=boolean
  Compare To Constant1 : simulink/Logic and Bit Operations/Compare To Constant  relop===  const=FALSE  OutDataTypeStr=boolean
  Compare To Constant2 : simulink/Logic and Bit Operations/Compare To Constant  relop===  const=TRUE  OutDataTypeStr=boolean
  Compare To Constant3 : simulink/Logic and Bit Operations/Compare To Constant  relop===  const=FALSE  OutDataTypeStr=boolean
  Logical Operator : Logic  Inputs=3  AllPortsSameDT=off  OutDataTypeStr=boolean
  Logical Operator1 : Logic  Inputs=3  AllPortsSameDT=off  OutDataTypeStr=boolean
  Unit Delay : UnitDelay  SampleTime=-1  HasFrameUpgradeWarning=on
  Unit Delay1 : UnitDelay  SampleTime=-1  HasFrameUpgradeWarning=on
  ABPFuncManeuAbor : Outport
  ABAFuncManeuAbor : Outport  Port=2
  wires:
  Logical Operator1/1 -> ABAFuncManeuAbor/1  "ABAFuncOptAbor"
  Logical Operator/1 -> ABPFuncManeuAbor/1  "ABPFuncOptAbor"
  ABAActive/1 -> Unit Delay1/1, Compare To Constant3/1
  Unit Delay1/1 -> Compare To Constant2/1
  ABATrigger/1 -> Logical Operator1/1
  Compare To Constant3/1 -> Logical Operator1/2
  Compare To Constant2/1 -> Logical Operator1/3
  Compare To Constant/1 -> Logical Operator/3
  Compare To Constant1/1 -> Logical Operator/2
  ABPTrigger/1 -> Logical Operator/1
  Unit Delay/1 -> Compare To Constant/1
  ABPActive/1 -> Compare To Constant1/1, Unit Delay/1

== system TosAeb/FcwAebJa_Step_sys/ALG_AEBSystem/ALG_AEBController/AEB_FuncImpManagementApp/FuncManeuArbCalc/BrkReadyManeuArbCalc/BrkReadyTriggerJudge ==
  breakReadyFuncImpArb : Inport
  ABPAvailable : Inport  Port=2
  ABAAvailable : Inport  Port=3
  longCollisionSeverity : Inport  Port=4
  pedCollisionSeverity : Inport  Port=5
  FCTB_ABA_Level : Inport  Port=6
  ABALvlCalc : Subsystem
  ABATriggerCalc : Subsystem
  ABPTriggerCalc : Subsystem
  Bus Selector : BusSelector  OutputSignals=prefillFuncImpArb,pedPrefillFuncImpArb,cycPrefillFuncImpArb,JA_vehPrefillFuncImpArb,JA_pedPrefillFuncImpArb,JA_cycPrefillFuncImpArb
  Bus Selector1 : BusSelector  OutputSignals=ABAFuncImpArb,pedABAFuncImpArb,FCTB_ABAFuncImpArb
  Bus Selector2 : BusSelector  OutputSignals=ABAFuncImpArb,pedABAFuncImpArb,FCTB_ABAFuncImpArb
  ABPTrigger : Outport
  ABATrigger : Outport  Port=2
  ABALvl : Outport  Port=3
  wires:
  FCTB_ABA_Level/1 -> ABALvlCalc/7(FCTB_ABA_Level)
  pedCollisionSeverity/1 -> ABALvlCalc/6(pedCollisionSeverity)
  longCollisionSeverity/1 -> ABALvlCalc/5(longCollisionSeverity)
  Bus Selector2/3 -> ABALvlCalc/4(FCTB_ABAFuncImpArb)  "<FCTB_ABAFuncImpArb>"
  Bus Selector2/2 -> ABALvlCalc/3(pedABAFuncImpArb)  "<pedABAFuncImpArb>"
  Bus Selector2/1 -> ABALvlCalc/2(ABAFuncImpArb)  "<ABAFuncImpArb>"
  Bus Selector1/2 -> ABATriggerCalc/3(pedABAFuncImpArb)  "<pedABAFuncImpArb>"
  Bus Selector1/3 -> ABATriggerCalc/4(FCTB_ABAFuncImpArb)  "<FCTB_ABAFuncImpArb>"
  Bus Selector1/1 -> ABATriggerCalc/2(ABAFuncImpArb)  "<ABAFuncImpArb>"
  Bus Selector/3 -> ABPTriggerCalc/4(cycPrefillFuncImpArb)  "<cycPrefillFuncImpArb>"
  Bus Selector/4 -> ABPTriggerCalc/5(JA_vehPrefillFuncImpArb)  "<JA_vehPrefillFuncImpArb>"
  Bus Selector/1 -> ABPTriggerCalc/2(prefillFuncImpArb)  "<prefillFuncImpArb>"
  Bus Selector/5 -> ABPTriggerCalc/6(JA_pedPrefillFuncImpArb)  "<JA_pedPrefillFuncImpArb>"
  Bus Selector/2 -> ABPTriggerCalc/3(pedPrefillFuncImpArb)  "<pedPrefillFuncImpArb>"
  Bus Selector/6 -> ABPTriggerCalc/7(JA_cycPedfillFuncImpArb)  "<JA_cycPrefillFuncImpArb>"
  ABALvlCalc/1(ABALvl) -> ABALvl/1
  ABATriggerCalc/1(ABATrigger) -> ABALvlCalc/1(ABATrigger), ABATrigger/1
  ABAAvailable/1 -> ABATriggerCalc/1(ABAAvailable)
  ABPTriggerCalc/1(ABPTrigger) -> ABPTrigger/1
  ABPAvailable/1 -> ABPTriggerCalc/1(ABPAvailable)
  breakReadyFuncImpArb/1 -> Bus Selector/1, Bus Selector1/1, Bus Selector2/1

== system TosAeb/FcwAebJa_Step_sys/ALG_AEBSystem/ALG_AEBController/AEB_FuncImpManagementApp/FuncManeuArbCalc/WarningManeuArbCalc/WarningAborCalc ==
  acuteWarningTrigger : Inport
  AWBActive : Inport  Port=2
  Compare To Constant : simulink/Logic and Bit Operations/Compare To Constant  relop===  const=1  OutDataTypeStr=boolean
  Compare To Constant1 : simulink/Logic and Bit Operations/Compare To Constant  relop===  const=0  OutDataTypeStr=boolean
  Logical Operator : Logic  Inputs=3  AllPortsSameDT=off  OutDataTypeStr=boolean
  Unit Delay : UnitDelay  SampleTime=-1  HasFrameUpgradeWarning=on
  acuteWarningFuncManeuAbor : Outport
  wires:
  Logical Operator/1 -> acuteWarningFuncManeuAbor/1
  Compare To Constant/1 -> Logical Operator/3
  Compare To Constant1/1 -> Logical Operator/2
  acuteWarningTrigger/1 -> Logical Operator/1
  Unit Delay/1 -> Compare To Constant/1
  AWBActive/1 -> Compare To Constant1/1, Unit Delay/1

== system TosAeb/FcwAebJa_Step_sys/ALG_AEBSystem/ALG_AEBController/AEB_FuncImpManagementApp/FuncManeuArbCalc/WarningManeuArbCalc/WarningTriggerCalc ==
  note: change the chart jerkleve logic&& ABA level
  AEBModStsFuncImpArb : Inport
  subjVel : Inport  Port=2
  Bus Selector : BusSelector  OutputSignals=warningFuncImpArb.latentWarningFuncImpArb
  Bus Selector1 : BusSelector  OutputSignals=warningFuncImpArb.pedPreWarningFuncImpArb,warningFuncImpArb.preWarningFuncImpArb
  Bus Selector2 : BusSelector  OutputSignals=warningFuncImpArb.acuteWarningFuncImpArb
  Compare To Constant{,1..3} (4 alike) : simulink/Logic and Bit Operations/Compare To Constant  relop===  const=ModSts.ActiveState  OutDataTypeStr=boolean
  Constant : Constant  Value=P_AEB_FIM_AcutewarningLvl1VehSpdMinThres
  Constant1 : Constant  Value=P_AEB_FIM_AcutewarningLvl2VehSpdMinThres
  Constant2 : Constant  Value=P_AEB_FIM_AcutewarningLvl3VehSpdMinThres
  Constant3 : Constant  Value=P_AEB_FIM_AcutewarningLvl4VehSpdMinThres
  Constant4 : Constant  Value=P_AEB_FIM_AcutewarningLvl4VehSpdMaxThres
  Logical Operator : Logic  Operator=OR  AllPortsSameDT=off  OutDataTypeStr=boolean
  acuteWarningLvlCalcFlow : Stateflow chart  TreatAsAtomicUnit=on
  latentWarningTrigger : Outport
  preWarningTrigger : Outport  Port=2
  acuteWarningTrigger : Outport  Port=3
  acuteWarningLvl : Outport  Port=4
  wires:
  Constant4/1 -> acuteWarningLvlCalcFlow/7(P_FIM_AcutewarningLvl4VehSpdMaxThres)
  Constant3/1 -> acuteWarningLvlCalcFlow/6(P_FIM_AcutewarningLvl4VehSpdMinThres)
  Constant2/1 -> acuteWarningLvlCalcFlow/5(P_FIM_AcutewarningLvl3VehSpdMinThres)
  Constant1/1 -> acuteWarningLvlCalcFlow/4(P_FIM_AcutewarningLvl2VehSpdMinThres)
  Constant/1 -> acuteWarningLvlCalcFlow/3(P_FIM_AcutewarningLvl1VehSpdMinThres)
  Logical Operator/1 -> preWarningTrigger/1
  Compare To Constant1/1 -> Logical Operator/1
  acuteWarningLvlCalcFlow/1(actuteWarningLvl) -> acuteWarningLvl/1
  Compare To Constant/1 -> latentWarningTrigger/1
  AEBModStsFuncImpArb/1 -> Bus Selector/1, Bus Selector1/1, Bus Selector2/1
  Bus Selector/1 -> Compare To Constant/1  "<latentWarningFuncImpArb>"
  subjVel/1 -> acuteWarningLvlCalcFlow/2(subjVel)
  Bus Selector2/1 -> Compare To Constant3/1  "<acuteWarningFuncImpArb>"
  Compare To Constant2/1 -> Logical Operator/2
  Bus Selector1/2 -> Compare To Constant2/1  "<preWarningFuncImpArb>"
  Bus Selector1/1 -> Compare To Constant1/1  "<pedPreWarningFuncImpArb>"
  Compare To Constant3/1 -> acuteWarningLvlCalcFlow/1(acuteWarningTrigger), acuteWarningTrigger/1

== system TosAeb/FcwAebJa_Step_sys/ALG_AEBSystem/ALG_AEBController/AEB_FuncImpManagementApp/FuncManeuArbCalc/brkFuncManeuArbCalc/AEBTriggerStateCalc ==
  AEBAvailable : Inport
  brkFuncImpArb : Inport  Port=2
  FctbIsNeedBrake : Inport  Port=3
  Bus Selector : BusSelector  OutputSignals=AEBpartFuncImpArb,cycAEBpartFuncImpArb,AEBfullFuncImpArb,JA_AEBfullFuncImpArb,cycAEBfullFuncImpArb,pedAEBfullFuncImpArb,EBAFuncImpArb,JA_PTW_AEBFuncImpArb,JA_cycAEBFuncImpArb,JA_pedAEBFuncImpArb,FCTBFuncImpArb
  Compare To Constant{,1..10} (11 alike) : simulink/Logic and Bit Operations/Compare To Constant  relop===  const=ModSts.ActiveState  OutDataTypeStr=boolean
  Logical Operator : Logic  Operator=OR  Inputs=11  AllPortsSameDT=off  OutDataTypeStr=boolean
  Logical Operator1 : Logic  AllPortsSameDT=off  OutDataTypeStr=boolean
  Logical Operator2 : Logic  AllPortsSameDT=off  OutDataTypeStr=boolean
  AEBTrigger : Outport
  wires:
  Logical Operator2/1 -> Logical Operator/11
  FctbIsNeedBrake/1 -> Logical Operator2/2
  Bus Selector/11 -> Compare To Constant10/1  "<FCTBFuncImpArb>"
  Bus Selector/10 -> Compare To Constant9/1  "<JA_pedAEBFuncImpArb>"
  Bus Selector/9 -> Compare To Constant8/1  "<JA_cycAEBFuncImpArb>"
  Bus Selector/8 -> Compare To Constant7/1  "<JA_PTW_AEBFuncImpArb>"
  brkFuncImpArb/1 -> Bus Selector/1
  Bus Selector/4 -> Compare To Constant3/1  "<JA_AEBfullFuncImpArb>"
  Bus Selector/7 -> Compare To Constant6/1  "<EBAFuncImpArb>"
  Compare To Constant6/1 -> Logical Operator/7
  AEBAvailable/1 -> Logical Operator1/1
  Compare To Constant10/1 -> Logical Operator2/1
  Compare To Constant/1 -> Logical Operator/1
  Compare To Constant4/1 -> Logical Operator/5
  Bus Selector/2 -> Compare To Constant1/1  "<cycAEBpartFuncImpArb>"
  Compare To Constant3/1 -> Logical Operator/4
  Bus Selector/1 -> Compare To Constant/1  "<AEBpartFuncImpArb>"
  Bus Selector/6 -> Compare To Constant5/1  "<pedAEBfullFuncImpArb>"
  Bus Selector/3 -> Compare To Constant2/1  "<AEBfullFuncImpArb>"
  Bus Selector/5 -> Compare To Constant4/1  "<cycAEBfullFuncImpArb>"
  Logical Operator1/1 -> AEBTrigger/1
  Compare To Constant5/1 -> Logical Operator/6
  Compare To Constant9/1 -> Logical Operator/10
  Compare To Constant7/1 -> Logical Operator/8
  Logical Operator/1 -> Logical Operator1/2
  Compare To Constant8/1 -> Logical Operator/9
  Compare To Constant2/1 -> Logical Operator/3
  Compare To Constant1/1 -> Logical Operator/2
